simulink model "ofdm_txrx_supermimo"  (Simulink 7.1)
settings: Solver=VariableStepDiscrete  SolverMode=SingleTasking  StartTime=0.0  StopTime=simOnly_simLength  RelTol=1e-3
PostLoadFcn:
  | ofdm_rx_supermimo_init
InitFcn:
  | ofdm_rx_supermimo_init
StartFcn:
  | ofdm_rx_supermimo_init
values from neighbouring files (symbols this model uses):
  Action0_Config = 0* 31*2^18 + 0*2^24 + PHY_AUTORESPONSE_REQ_GOODHDR + PHY_AUTORESPONSE_REQ_GOODPKT + PHY_AUTORESPONSE_REQ_FLAGA    [ofdm_tx_supermimo_init.m]
  Action1_Config = 0* 62*2^18 + PHY_AUTORESPONSE_REQ_GOODHDR + PHY_AUTORESPONSE_REQ_GOODPKT    [ofdm_tx_supermimo_init.m]
  Action2_Config = 0    [ofdm_tx_supermimo_init.m]
  Action3_Config = 0    [ofdm_tx_supermimo_init.m]
  Action4_Config = 0    [ofdm_tx_supermimo_init.m]
  Action5_Config = 0    [ofdm_tx_supermimo_init.m]
  ActionID_AFTransmit = 31    [ofdm_tx_supermimo_init.m]
  ActionID_Disabled = 0    [ofdm_tx_supermimo_init.m]
  ActionID_SetFlagA = 62    [ofdm_tx_supermimo_init.m]
  ActionID_SetFlagB = 61    [ofdm_tx_supermimo_init.m]
  BER_RAM_init_size = 2048    [ofdm_tx_supermimo_init.m]
  CPLength = 16    [ofdm_tx_supermimo_init.m]
  longCorr_coef_i = [-4*real(LTS) 0]    [ofdm_rx_supermimo_init.m]
  longCorr_coef_q = [4*imag(LTS) 0]    [ofdm_rx_supermimo_init.m]
  Match0_Config = 0    [ofdm_tx_supermimo_init.m]
  Match1_Config = 0    [ofdm_tx_supermimo_init.m]
  Match2_Config = 0    [ofdm_tx_supermimo_init.m]
  Match3_Config = 0    [ofdm_tx_supermimo_init.m]
  Match4_Config = 0    [ofdm_tx_supermimo_init.m]
  Match5_Config = 0    [ofdm_tx_supermimo_init.m]
  Match6_Config = 0    [ofdm_tx_supermimo_init.m]
  Match7_Config = 0    [ofdm_tx_supermimo_init.m]
  max_CP_length = 16    [ofdm_tx_supermimo_init.m]
  max_num_baseRateSymbols = 31    [ofdm_tx_supermimo_init.m]
  max_num_subcarriers = 64    [ofdm_tx_supermimo_init.m]
  max_num_trainingSymbols = 15    [ofdm_tx_supermimo_init.m]
  max_numBytes = 16384    [ofdm_tx_supermimo_init.m]
  max_OFDM_symbols = 511    [ofdm_tx_supermimo_init.m]
  modConstellation_qam16 = .75*[1 3 -1 -3]./3    [ofdm_tx_supermimo_init.m]
  modConstellation_qam64 = 0.875*[3 1 5 7 -3 -1 -5 -7]./7    [ofdm_tx_supermimo_init.m]
  modConstellation_qpsk = [1 -1]./sqrt(2)    [ofdm_tx_supermimo_init.m]
  numBaseRateSymbols = ceil(numHeaderBytes / numBytes_BaseRateOFDMSymbol)    [ofdm_tx_supermimo_init.m]
  numSubcarriers = 64    [ofdm_tx_supermimo_init.m]
  numTrainingSymbols = 2    [ofdm_tx_supermimo_init.m]
  pilot_indicies = 7 + ( (64-21) * 2^8) + ( (64-7) * 2^16) + (21 * 2^24)    (= 356068103)    [ofdm_tx_supermimo_init.m]
  pilotValues = hex2dec('A57D') + (2^16 * hex2dec('5A82'))    [ofdm_tx_supermimo_init.m]
  pkt_crossCorr_thresh = 0.7    [ofdm_rx_supermimo_init.m]
  pkt_energy_thresh = 0    [ofdm_rx_supermimo_init.m]
  pktByteNums = numHeaderBytes +  				byteIndex_numPayloadBytes(1)*2^8 +  				byteIndex_numPayloadBytes(2)*2^16 +  				byteIndex_simpleDynModMasks * 2^24    [ofdm_rx_supermimo_init.m]
  PktDet_Delay = 58+32 +      Symbol_Timing_Offset*2^7 +      0*2^16 +      (117* 2^24)    (= 1962935642)    [ofdm_rx_supermimo_init.m]
  pktTiming_count_payloadStart = pktTiming_count_lsStart + pktTiming_ls_num*pktTiming_ls_length    [ofdm_rx_supermimo_init.m]
  preamble = preamble_scale*[shortsyms_10 longsyms_2]    [ofdm_tx_supermimo_init.m]
  preamble_ROM = preamble_I_ROM + preamble_Q_ROM*2^16    [ofdm_tx_supermimo_init.m]
  RAM_init_size = max_numBytes    (= 16384)    [ofdm_tx_supermimo_init.m]
  reg_AF_Tx_Blanking = (320+80) + ( (320+80+79)*2^16)    (= 31392144)    [ofdm_rx_supermimo_init.m]
  reg_AF_Tx_Scaling = 2944    [ofdm_rx_supermimo_init.m]
  reg_Interrupt_PktBuf_Control = reg_InterruptControl +  	1 * 2^16 +  	0 * 2^24    (= 65656)    [ofdm_rx_supermimo_init.m]
  reg_PilotCalcParams = (4 * 2^16) + (minPilotChanMag)    (= 262184.96)    [ofdm_rx_supermimo_init.m]
  regRx_chanEst_minMag = ((0.5)*2^15) + ((0.5 * 2^15)*2^16)    (= 1073758208)    [ofdm_rx_supermimo_init.m]
  regRx_coarseCalcCorrection = round((1.3e-3 - 1.2982e-3)*2^32)    [ofdm_rx_supermimo_init.m]
  regRx_pilotCalcCorrection = round(1.00115 * 2^31)    [ofdm_rx_supermimo_init.m]
  regRx_preCFOoptions = 0 * 2^0 +  	1 * 2^1 +  	0    (= 2)    [ofdm_rx_supermimo_init.m]
  rx_controlBits = 1 * 2  		 + 1 * 4  		 + 0 * 8  		 + 1 * 16 * rx_SISO_Mode  		 + 1 * 32  		 + 1 * 64  		 + 1 * 128  		 + 1 * 256  		 + 1 * 512  		 + 1 * 1024  		 + 1 * 4096  		 + 0 * 16384  		 + 0 * 32768  		 + 1 * 65536  		 + 0 * 131072  		 + 0 * 262144  		 + 1 * 524288  		 + 1 * 1048576 * tx_Alamouti_Mode  		 + 0 * 2097152           + 1 * 33554432  		 + 0 * 4194304  		 + 0 * 8388608  		 + 0 * 16777216  		 + 1 *  ...(+49 chars)    [ofdm_rx_supermimo_init.m]
  Rx_PktDet_LongCorr_Params = LongCorrCounterLoad + 0*2^8 + 0*2^16 + 255*2^24    (= 4278190325)    [ofdm_rx_supermimo_init.m]
  Rx_PktDet_LongCorrThresholds = 8000    [ofdm_rx_supermimo_init.m]
  rx_postEq_scaling = round(rxScaling*2^11) * (1 + 2^16)    [ofdm_rx_supermimo_init.m]
  RxDataScrambling_Seq = [40 198 78 63 82 173 102 245 48 111 172 115 147 230 216 93 72 65 62 2 205 242 122 90 128 83 105 97 73 10 5 252]    [ofdm_tx_supermimo_init.m]
  RxReg_FixedPktLen = 0    [ofdm_rx_supermimo_init.m]
  shortSymbol_time = shortSymbol_time(1:16).'    [ofdm_tx_supermimo_init.m]
  simOnly_numSamples = length(preamble)+( (numSubcarriers+CPLength)*(numTrainingSymbols + numBaseRateSymbols + numFullRateSymbols/2) )    [ofdm_tx_supermimo_init.m]
  train = [train train]    [ofdm_tx_supermimo_init.m]
  tx_controlBits = (antB_preambleShift * 2^4) + (2^1 * tx_Alamouti_Mode) +  	(2^0 * tx_SISO_Mode) + 4*0 + 8*1 + 0*256 + 512*0 + 1024*0 +  	0*hex2dec('1000') + 0*hex2dec('2000') + 0*hex2dec('4000') +  	1*hex2dec('8000') + 1*hex2dec('10000') + hex2dec('20000') +  	16*hex2dec('100000')    [ofdm_tx_supermimo_init.m]
  tx_delays = (50*2^0) + (0*2^8) + (30*2^12)    (= 122930)    [ofdm_tx_supermimo_init.m]
  tx_scaling = (3072) + (12288 * 2^16)    (= 805309440)    [ofdm_tx_supermimo_init.m]
  TxDataScrambling_Seq = [40 198 78 63 82 173 102 245 48 111 172 115 147 230 216 93 72 65 62 2 205 242 122 90 128 83 105 97 73 10 5 252]    [ofdm_tx_supermimo_init.m]
  txfilt_firpm = 0.95*txfilt_firpm./(max(txfilt_firpm))    [ofdm_tx_supermimo_init.m]
  txfilt_intfilt = [0 intfilt(4,4,.8)]    [ofdm_tx_supermimo_init.m]
  txReg_symbolCounts = (2^8 *numBaseRateSymbols ) + numTrainingSymbols    [ofdm_tx_supermimo_init.m]
  TxRx_FFTScaling = (3 * 2^0) +  	(2 * 2^2) +  	(1 * 2^4) +  	(1 * 2^6) +  	(1 * 2^8) +  	(0 * 2^10)    (= 347)    [ofdm_tx_supermimo_init.m]
undefined symbols (not defined in the file or in its folder): AntA, AntB, BaseRate, Down, gcbh, numBuffs, numBytes, Up, xlBlockLoadCallback, xlGetSimulinkPeriod, xlipgetpartsetting
libraries: xbsIndex_r4 x6035, simulink x4, dspsigops x4, commchan3 x2, dspsrcs4 x2, commrflib2 x2
(part 47 of 61)

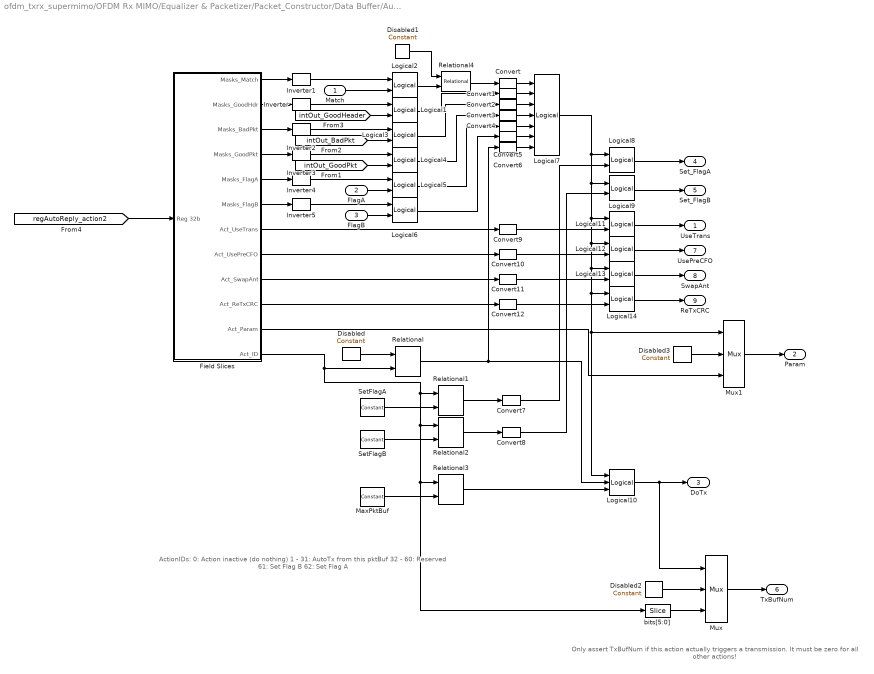
== system ofdm_txrx_supermimo/OFDM Rx MIMO/Equalizer & Packetizer/Packet_Constructor/Data Buffer/AutoResponse Processing/Action Decoding/Action2 ==
  note: ActionIDs:
      | 0: Action inactive (do nothing)
      | 1 - 31: AutoTx from this pktBuf
      | 32 - 60: Reserved
      | 61: Set Flag B
      | 62: Set Flag A
  note: Only assert TxBufNum if this action
      | actually triggers a transmission. It must
      | be zero for all other actions!
  Match : Inport
  FlagA : Inport  Port=2  OutDataType=sfix(16)  OutScaling=2^0
  FlagB : Inport  Port=3  OutDataType=sfix(16)  OutScaling=2^0
  Convert{,1..12} (13 alike) : xbsIndex_r4/Convert  arith_type=Boolean  n_bits=16  bin_pt=14  quantization=Truncate  overflow=Wrap  latency=0  dbl_ovrd=off  pipeline=off  block_type=convert
      settings: block_version=10.1.3
  Disabled : xbsIndex_r4/Constant  arith_type=Unsigned  const=ActionID_Disabled  n_bits=6  bin_pt=0  explicit_period=off  period=1  equ=P=C  opselect=C  inp2=PCIN>>17  opr=+  inp1=P  carry=CIN  dbl_ovrd=off  block_type=constant
  Disabled1 : xbsIndex_r4/Constant  arith_type=Unsigned  const=2^8-1  n_bits=8  bin_pt=0  explicit_period=off  period=1  equ=P=C  opselect=C  inp2=PCIN>>17  opr=+  inp1=P  carry=CIN  dbl_ovrd=off  block_type=constant
  Disabled2 : xbsIndex_r4/Constant  arith_type=Unsigned  const=0  n_bits=5  bin_pt=0  explicit_period=off  period=1  equ=P=C  opselect=C  inp2=PCIN>>17  opr=+  inp1=P  carry=CIN  dbl_ovrd=off  block_type=constant
  Disabled3 : xbsIndex_r4/Constant  arith_type=Unsigned  const=0  n_bits=8  bin_pt=0  explicit_period=off  period=1  equ=P=C  opselect=C  inp2=PCIN>>17  opr=+  inp1=P  carry=CIN  dbl_ovrd=off  block_type=constant
  Field Slices : Subsystem
  Inverter{,1..5} (6 alike) : xbsIndex_r4/Inverter  en=off  latency=0  dbl_ovrd=off  block_type=inv
  Logical{1..6} (6 alike) : xbsIndex_r4/Logical  logical_function=OR  inputs=2  en=off  latency=0  precision=Full  arith_type=Unsigned  n_bits=16  bin_pt=0  align_bp=on  dbl_ovrd=off  block_type=logical
  Logical10 : xbsIndex_r4/Logical  logical_function=AND  inputs=3  en=off  latency=0  precision=Full  arith_type=Unsigned  n_bits=16  bin_pt=0  align_bp=on  dbl_ovrd=off  block_type=logical
  Logical{8..9,11..14} (6 alike) : xbsIndex_r4/Logical  logical_function=AND  inputs=2  en=off  latency=0  precision=Full  arith_type=Unsigned  n_bits=16  bin_pt=0  align_bp=on  dbl_ovrd=off  block_type=logical
  Logical7 : xbsIndex_r4/Logical  logical_function=AND  inputs=7  en=off  latency=0  precision=Full  arith_type=Unsigned  n_bits=16  bin_pt=0  align_bp=on  dbl_ovrd=off  block_type=logical
  MaxPktBuf : xbsIndex_r4/Constant  arith_type=Unsigned  const=32  n_bits=6  bin_pt=0  explicit_period=off  period=1  equ=P=C  opselect=C  inp2=PCIN>>17  opr=+  inp1=P  carry=CIN  dbl_ovrd=off  block_type=constant
  Mux : xbsIndex_r4/Mux  inputs=2  en=off  latency=0  precision=Full  arith_type=Unsigned  n_bits=16  bin_pt=14  quantization=Truncate  overflow=Wrap  dbl_ovrd=off  block_type=mux
  Mux1 : xbsIndex_r4/Mux  inputs=2  en=off  latency=0  precision=Full  arith_type=Unsigned  n_bits=16  bin_pt=14  quantization=Truncate  overflow=Wrap  dbl_ovrd=off  block_type=mux
  Relational : xbsIndex_r4/Relational  mode=a!=b  en=off  latency=0  dbl_ovrd=off  block_type=relational
      settings: block_version=10.1.3
  Relational1 : xbsIndex_r4/Relational  mode=a=b  en=off  latency=0  dbl_ovrd=off  block_type=relational
      settings: block_version=10.1.3
  Relational2 : xbsIndex_r4/Relational  mode=a=b  en=off  latency=0  dbl_ovrd=off  block_type=relational
      settings: block_version=10.1.3
  Relational3 : xbsIndex_r4/Relational  mode=a<b  en=off  latency=0  dbl_ovrd=off  block_type=relational
      settings: block_version=10.1.3
  Relational4 : xbsIndex_r4/Relational  mode=a=b  en=off  latency=0  dbl_ovrd=off  block_type=relational
      settings: block_version=10.1.3
  SetFlagA : xbsIndex_r4/Constant  arith_type=Unsigned  const=ActionID_SetFlagA  n_bits=6  bin_pt=0  explicit_period=off  period=1  equ=P=C  opselect=C  inp2=PCIN>>17  opr=+  inp1=P  carry=CIN  dbl_ovrd=off  block_type=constant
  SetFlagB : xbsIndex_r4/Constant  arith_type=Unsigned  const=ActionID_SetFlagB  n_bits=6  bin_pt=0  explicit_period=off  period=1  equ=P=C  opselect=C  inp2=PCIN>>17  opr=+  inp1=P  carry=CIN  dbl_ovrd=off  block_type=constant
  bits[5:0] : xbsIndex_r4/Slice  nbits=5  boolean_output=off  mode=Lower Bit Location + Width  bit1=0  base1=MSB of Input  bit0=0  base0=LSB of Input  dbl_ovrd=off  block_type=slice
      settings: infoedit="Extracts a given range of bits from each input sample and presents it at the output.  The output type is ordinarily unsigned with binary point at zero, but can be Boolean when the slice is one bit wide.<P><P>Hardware notes: In hardware this block costs nothing."  block_version=VER_STRING_GOES_HERE
  UseTrans : Outport
  Param : Outport  Port=2
  DoTx : Outport  Port=3
  Set_FlagA : Outport  Port=4
  Set_FlagB : Outport  Port=5
  TxBufNum : Outport  Port=6
  UsePreCFO : Outport  Port=7
  SwapAnt : Outport  Port=8
  ReTxCRC : Outport  Port=9
  wires:
  Mux/1 -> TxBufNum/1
  Disabled2/1 -> Mux/2
  bits[5:0]/1 -> Mux/3
  FlagB/1 -> Logical6/2
  FlagA/1 -> Logical5/2
  Field Slices/11(Act_Param) -> Mux1/3
  Field Slices/7(Act_UseTrans) -> Convert9/1
  Match/1 -> Logical2/2
  Logical10/1 -> Mux/1, DoTx/1
  Relational3/1 -> Logical10/3
  Relational2/1 -> Convert8/1
  Relational1/1 -> Convert7/1
  Logical9/1 -> Set_FlagB/1
  Logical7/1 -> Logical9/1, Logical11/1, Logical12/1, Logical13/1, Logical10/1, Mux1/1, Logical14/1, Logical8/1
  Logical8/1 -> Set_FlagA/1
  Relational/1 -> Convert6/1, Logical10/2
  Logical6/1 -> Convert5/1
  Logical5/1 -> Convert4/1
  Logical4/1 -> Convert3/1
  Logical3/1 -> Convert2/1
  Logical1/1 -> Convert1/1
  Logical2/1 -> Relational4/2
  MaxPktBuf/1 -> Relational3/2
  SetFlagB/1 -> Relational2/2
  SetFlagA/1 -> Relational1/2
  Field Slices/12(Act_ID) -> Relational2/1, Relational3/1, bits[5:0]/1, Relational1/1, Relational/2
  Inverter5/1 -> Logical6/1
  Field Slices/6(Masks_FlagB) -> Inverter5/1
  Inverter4/1 -> Logical5/1
  Field Slices/5(Masks_FlagA) -> Inverter4/1
  Field Slices/4(Masks_GoodPkt) -> Inverter3/1
  [intOut_GoodPkt] -> Logical4/2
  Inverter3/1 -> Logical4/1
  [intOut_BadPkt] -> Logical3/2
  Inverter2/1 -> Logical3/1
  Field Slices/3(Masks_BadPkt) -> Inverter2/1
  Inverter1/1 -> Logical2/1
  [intOut_GoodHeader] -> Logical1/2
  Inverter/1 -> Logical1/1
  Field Slices/2(Masks_GoodHdr) -> Inverter/1
  Field Slices/1(Masks_Match) -> Inverter1/1
  [regAutoReply_action2] -> Field Slices/1(Reg 32b)
  Convert/1 -> Logical7/1
  Convert1/1 -> Logical7/2
  Convert2/1 -> Logical7/3
  Convert3/1 -> Logical7/4
  Convert4/1 -> Logical7/5
  Convert5/1 -> Logical7/6
  Convert6/1 -> Logical7/7
  Convert7/1 -> Logical8/2
  Convert8/1 -> Logical9/2
  Relational4/1 -> Convert/1
  Disabled1/1 -> Relational4/1
  Logical11/1 -> UseTrans/1
  Mux1/1 -> Param/1
  Disabled3/1 -> Mux1/2
  Convert9/1 -> Logical11/2
  Field Slices/8(Act_UsePreCFO) -> Convert10/1
  Convert10/1 -> Logical12/2
  Logical12/1 -> UsePreCFO/1
  Logical13/1 -> SwapAnt/1
  Field Slices/9(Act_SwapAnt) -> Convert11/1
  Convert11/1 -> Logical13/2
  Disabled/1 -> Relational/1
  Field Slices/10(Act_ReTxCRC) -> Convert12/1
  Convert12/1 -> Logical14/2
  Logical14/1 -> ReTxCRC/1

== system ofdm_txrx_supermimo/OFDM Rx MIMO/Equalizer & Packetizer/Packet_Constructor/Data Buffer/AutoResponse Processing/Action Decoding/Action3 ==
  note: Only assert TxBufNum if this action
      | actually triggers a transmission. It must
      | be zero for all other actions!
  note: ActionIDs:
      | 0: Action inactive (do nothing)
      | 1 - 31: AutoTx from this pktBuf
      | 32 - 60: Reserved
      | 61: Set Flag B
      | 62: Set Flag A
  Match : Inport
  FlagA : Inport  Port=2  OutDataType=sfix(16)  OutScaling=2^0
  FlagB : Inport  Port=3  OutDataType=sfix(16)  OutScaling=2^0
  Convert{,1..12} (13 alike) : xbsIndex_r4/Convert  arith_type=Boolean  n_bits=16  bin_pt=14  quantization=Truncate  overflow=Wrap  latency=0  dbl_ovrd=off  pipeline=off  block_type=convert
      settings: block_version=10.1.3
  Disabled : xbsIndex_r4/Constant  arith_type=Unsigned  const=ActionID_Disabled  n_bits=6  bin_pt=0  explicit_period=off  period=1  equ=P=C  opselect=C  inp2=PCIN>>17  opr=+  inp1=P  carry=CIN  dbl_ovrd=off  block_type=constant
  Disabled1 : xbsIndex_r4/Constant  arith_type=Unsigned  const=2^8-1  n_bits=8  bin_pt=0  explicit_period=off  period=1  equ=P=C  opselect=C  inp2=PCIN>>17  opr=+  inp1=P  carry=CIN  dbl_ovrd=off  block_type=constant
  Disabled2 : xbsIndex_r4/Constant  arith_type=Unsigned  const=0  n_bits=5  bin_pt=0  explicit_period=off  period=1  equ=P=C  opselect=C  inp2=PCIN>>17  opr=+  inp1=P  carry=CIN  dbl_ovrd=off  block_type=constant
  Disabled3 : xbsIndex_r4/Constant  arith_type=Unsigned  const=0  n_bits=8  bin_pt=0  explicit_period=off  period=1  equ=P=C  opselect=C  inp2=PCIN>>17  opr=+  inp1=P  carry=CIN  dbl_ovrd=off  block_type=constant
  Field Slices : Subsystem
  Inverter{,1..5} (6 alike) : xbsIndex_r4/Inverter  en=off  latency=0  dbl_ovrd=off  block_type=inv
  Logical{1..6} (6 alike) : xbsIndex_r4/Logical  logical_function=OR  inputs=2  en=off  latency=0  precision=Full  arith_type=Unsigned  n_bits=16  bin_pt=0  align_bp=on  dbl_ovrd=off  block_type=logical
  Logical10 : xbsIndex_r4/Logical  logical_function=AND  inputs=3  en=off  latency=0  precision=Full  arith_type=Unsigned  n_bits=16  bin_pt=0  align_bp=on  dbl_ovrd=off  block_type=logical
  Logical{8..9,11..14} (6 alike) : xbsIndex_r4/Logical  logical_function=AND  inputs=2  en=off  latency=0  precision=Full  arith_type=Unsigned  n_bits=16  bin_pt=0  align_bp=on  dbl_ovrd=off  block_type=logical
  Logical7 : xbsIndex_r4/Logical  logical_function=AND  inputs=7  en=off  latency=0  precision=Full  arith_type=Unsigned  n_bits=16  bin_pt=0  align_bp=on  dbl_ovrd=off  block_type=logical
  MaxPktBuf : xbsIndex_r4/Constant  arith_type=Unsigned  const=32  n_bits=6  bin_pt=0  explicit_period=off  period=1  equ=P=C  opselect=C  inp2=PCIN>>17  opr=+  inp1=P  carry=CIN  dbl_ovrd=off  block_type=constant
  Mux : xbsIndex_r4/Mux  inputs=2  en=off  latency=0  precision=Full  arith_type=Unsigned  n_bits=16  bin_pt=14  quantization=Truncate  overflow=Wrap  dbl_ovrd=off  block_type=mux
  Mux1 : xbsIndex_r4/Mux  inputs=2  en=off  latency=0  precision=Full  arith_type=Unsigned  n_bits=16  bin_pt=14  quantization=Truncate  overflow=Wrap  dbl_ovrd=off  block_type=mux
  Relational : xbsIndex_r4/Relational  mode=a!=b  en=off  latency=0  dbl_ovrd=off  block_type=relational
      settings: block_version=10.1.3
  Relational1 : xbsIndex_r4/Relational  mode=a=b  en=off  latency=0  dbl_ovrd=off  block_type=relational
      settings: block_version=10.1.3
  Relational2 : xbsIndex_r4/Relational  mode=a=b  en=off  latency=0  dbl_ovrd=off  block_type=relational
      settings: block_version=10.1.3
  Relational3 : xbsIndex_r4/Relational  mode=a<b  en=off  latency=0  dbl_ovrd=off  block_type=relational
      settings: block_version=10.1.3
  Relational4 : xbsIndex_r4/Relational  mode=a=b  en=off  latency=0  dbl_ovrd=off  block_type=relational
      settings: block_version=10.1.3
  SetFlagA : xbsIndex_r4/Constant  arith_type=Unsigned  const=ActionID_SetFlagA  n_bits=6  bin_pt=0  explicit_period=off  period=1  equ=P=C  opselect=C  inp2=PCIN>>17  opr=+  inp1=P  carry=CIN  dbl_ovrd=off  block_type=constant
  SetFlagB : xbsIndex_r4/Constant  arith_type=Unsigned  const=ActionID_SetFlagB  n_bits=6  bin_pt=0  explicit_period=off  period=1  equ=P=C  opselect=C  inp2=PCIN>>17  opr=+  inp1=P  carry=CIN  dbl_ovrd=off  block_type=constant
  bits[5:0] : xbsIndex_r4/Slice  nbits=5  boolean_output=off  mode=Lower Bit Location + Width  bit1=0  base1=MSB of Input  bit0=0  base0=LSB of Input  dbl_ovrd=off  block_type=slice
      settings: infoedit="Extracts a given range of bits from each input sample and presents it at the output.  The output type is ordinarily unsigned with binary point at zero, but can be Boolean when the slice is one bit wide.<P><P>Hardware notes: In hardware this block costs nothing."  block_version=VER_STRING_GOES_HERE
  UseTrans : Outport
  Param : Outport  Port=2
  DoTx : Outport  Port=3
  Set_FlagA : Outport  Port=4
  Set_FlagB : Outport  Port=5
  TxBufNum : Outport  Port=6
  UsePreCFO : Outport  Port=7
  SwapAnt : Outport  Port=8
  ReTxCRC : Outport  Port=9
  wires:
  Logical14/1 -> ReTxCRC/1
  Convert12/1 -> Logical14/2
  Field Slices/10(Act_ReTxCRC) -> Convert12/1
  Disabled/1 -> Relational/1
  Convert11/1 -> Logical13/2
  Field Slices/9(Act_SwapAnt) -> Convert11/1
  Logical13/1 -> SwapAnt/1
  Logical12/1 -> UsePreCFO/1
  Convert10/1 -> Logical12/2
  Field Slices/8(Act_UsePreCFO) -> Convert10/1
  Convert9/1 -> Logical11/2
  Disabled3/1 -> Mux1/2
  Mux1/1 -> Param/1
  Logical11/1 -> UseTrans/1
  Disabled1/1 -> Relational4/1
  Relational4/1 -> Convert/1
  Convert8/1 -> Logical9/2
  Convert7/1 -> Logical8/2
  Convert6/1 -> Logical7/7
  Convert5/1 -> Logical7/6
  Convert4/1 -> Logical7/5
  Convert3/1 -> Logical7/4
  Convert2/1 -> Logical7/3
  Convert1/1 -> Logical7/2
  Convert/1 -> Logical7/1
  [regAutoReply_action3] -> Field Slices/1(Reg 32b)
  Field Slices/1(Masks_Match) -> Inverter1/1
  Field Slices/2(Masks_GoodHdr) -> Inverter/1
  Inverter/1 -> Logical1/1
  [intOut_GoodHeader] -> Logical1/2
  Inverter1/1 -> Logical2/1
  Field Slices/3(Masks_BadPkt) -> Inverter2/1
  Inverter2/1 -> Logical3/1
  [intOut_BadPkt] -> Logical3/2
  Inverter3/1 -> Logical4/1
  [intOut_GoodPkt] -> Logical4/2
  Field Slices/4(Masks_GoodPkt) -> Inverter3/1
  Field Slices/5(Masks_FlagA) -> Inverter4/1
  Inverter4/1 -> Logical5/1
  Field Slices/6(Masks_FlagB) -> Inverter5/1
  Inverter5/1 -> Logical6/1
  Field Slices/12(Act_ID) -> Relational/2, Relational1/1, bits[5:0]/1, Relational3/1, Relational2/1
  SetFlagA/1 -> Relational1/2
  SetFlagB/1 -> Relational2/2
  MaxPktBuf/1 -> Relational3/2
  Logical2/1 -> Relational4/2
  Logical1/1 -> Convert1/1
  Logical3/1 -> Convert2/1
  Logical4/1 -> Convert3/1
  Logical5/1 -> Convert4/1
  Logical6/1 -> Convert5/1
  Relational/1 -> Logical10/2, Convert6/1
  Logical8/1 -> Set_FlagA/1
  Logical7/1 -> Logical8/1, Logical14/1, Mux1/1, Logical10/1, Logical13/1, Logical12/1, Logical11/1, Logical9/1
  Logical9/1 -> Set_FlagB/1
  Relational1/1 -> Convert7/1
  Relational2/1 -> Convert8/1
  Relational3/1 -> Logical10/3
  Logical10/1 -> DoTx/1, Mux/1
  Match/1 -> Logical2/2
  Field Slices/7(Act_UseTrans) -> Convert9/1
  Field Slices/11(Act_Param) -> Mux1/3
  FlagA/1 -> Logical5/2
  FlagB/1 -> Logical6/2
  bits[5:0]/1 -> Mux/3
  Disabled2/1 -> Mux/2
  Mux/1 -> TxBufNum/1

== system ofdm_txrx_supermimo/OFDM Rx MIMO/Equalizer & Packetizer/Packet_Constructor/Data Buffer/AutoResponse Processing/Action Decoding/Action4 ==
  note: ActionIDs:
      | 0: Action inactive (do nothing)
      | 1 - 31: AutoTx from this pktBuf
      | 32 - 60: Reserved
      | 61: Set Flag B
      | 62: Set Flag A
  note: Only assert TxBufNum if this action
      | actually triggers a transmission. It must
      | be zero for all other actions!
  Match : Inport
  FlagA : Inport  Port=2  OutDataType=sfix(16)  OutScaling=2^0
  FlagB : Inport  Port=3  OutDataType=sfix(16)  OutScaling=2^0
  Convert{,1..12} (13 alike) : xbsIndex_r4/Convert  arith_type=Boolean  n_bits=16  bin_pt=14  quantization=Truncate  overflow=Wrap  latency=0  dbl_ovrd=off  pipeline=off  block_type=convert
      settings: block_version=10.1.3
  Disabled : xbsIndex_r4/Constant  arith_type=Unsigned  const=ActionID_Disabled  n_bits=6  bin_pt=0  explicit_period=off  period=1  equ=P=C  opselect=C  inp2=PCIN>>17  opr=+  inp1=P  carry=CIN  dbl_ovrd=off  block_type=constant
  Disabled1 : xbsIndex_r4/Constant  arith_type=Unsigned  const=2^8-1  n_bits=8  bin_pt=0  explicit_period=off  period=1  equ=P=C  opselect=C  inp2=PCIN>>17  opr=+  inp1=P  carry=CIN  dbl_ovrd=off  block_type=constant
  Disabled2 : xbsIndex_r4/Constant  arith_type=Unsigned  const=0  n_bits=5  bin_pt=0  explicit_period=off  period=1  equ=P=C  opselect=C  inp2=PCIN>>17  opr=+  inp1=P  carry=CIN  dbl_ovrd=off  block_type=constant
  Disabled3 : xbsIndex_r4/Constant  arith_type=Unsigned  const=0  n_bits=8  bin_pt=0  explicit_period=off  period=1  equ=P=C  opselect=C  inp2=PCIN>>17  opr=+  inp1=P  carry=CIN  dbl_ovrd=off  block_type=constant
  Field Slices : Subsystem
  Inverter{,1..5} (6 alike) : xbsIndex_r4/Inverter  en=off  latency=0  dbl_ovrd=off  block_type=inv
  Logical{1..6} (6 alike) : xbsIndex_r4/Logical  logical_function=OR  inputs=2  en=off  latency=0  precision=Full  arith_type=Unsigned  n_bits=16  bin_pt=0  align_bp=on  dbl_ovrd=off  block_type=logical
  Logical10 : xbsIndex_r4/Logical  logical_function=AND  inputs=3  en=off  latency=0  precision=Full  arith_type=Unsigned  n_bits=16  bin_pt=0  align_bp=on  dbl_ovrd=off  block_type=logical
  Logical{8..9,11..14} (6 alike) : xbsIndex_r4/Logical  logical_function=AND  inputs=2  en=off  latency=0  precision=Full  arith_type=Unsigned  n_bits=16  bin_pt=0  align_bp=on  dbl_ovrd=off  block_type=logical
  Logical7 : xbsIndex_r4/Logical  logical_function=AND  inputs=7  en=off  latency=0  precision=Full  arith_type=Unsigned  n_bits=16  bin_pt=0  align_bp=on  dbl_ovrd=off  block_type=logical
  MaxPktBuf : xbsIndex_r4/Constant  arith_type=Unsigned  const=32  n_bits=6  bin_pt=0  explicit_period=off  period=1  equ=P=C  opselect=C  inp2=PCIN>>17  opr=+  inp1=P  carry=CIN  dbl_ovrd=off  block_type=constant
  Mux : xbsIndex_r4/Mux  inputs=2  en=off  latency=0  precision=Full  arith_type=Unsigned  n_bits=16  bin_pt=14  quantization=Truncate  overflow=Wrap  dbl_ovrd=off  block_type=mux
  Mux1 : xbsIndex_r4/Mux  inputs=2  en=off  latency=0  precision=Full  arith_type=Unsigned  n_bits=16  bin_pt=14  quantization=Truncate  overflow=Wrap  dbl_ovrd=off  block_type=mux
  Relational : xbsIndex_r4/Relational  mode=a!=b  en=off  latency=0  dbl_ovrd=off  block_type=relational
      settings: block_version=10.1.3
  Relational1 : xbsIndex_r4/Relational  mode=a=b  en=off  latency=0  dbl_ovrd=off  block_type=relational
      settings: block_version=10.1.3
  Relational2 : xbsIndex_r4/Relational  mode=a=b  en=off  latency=0  dbl_ovrd=off  block_type=relational
      settings: block_version=10.1.3
  Relational3 : xbsIndex_r4/Relational  mode=a<b  en=off  latency=0  dbl_ovrd=off  block_type=relational
      settings: block_version=10.1.3
  Relational4 : xbsIndex_r4/Relational  mode=a=b  en=off  latency=0  dbl_ovrd=off  block_type=relational
      settings: block_version=10.1.3
  SetFlagA : xbsIndex_r4/Constant  arith_type=Unsigned  const=ActionID_SetFlagA  n_bits=6  bin_pt=0  explicit_period=off  period=1  equ=P=C  opselect=C  inp2=PCIN>>17  opr=+  inp1=P  carry=CIN  dbl_ovrd=off  block_type=constant
  SetFlagB : xbsIndex_r4/Constant  arith_type=Unsigned  const=ActionID_SetFlagB  n_bits=6  bin_pt=0  explicit_period=off  period=1  equ=P=C  opselect=C  inp2=PCIN>>17  opr=+  inp1=P  carry=CIN  dbl_ovrd=off  block_type=constant
  bits[5:0] : xbsIndex_r4/Slice  nbits=5  boolean_output=off  mode=Lower Bit Location + Width  bit1=0  base1=MSB of Input  bit0=0  base0=LSB of Input  dbl_ovrd=off  block_type=slice
      settings: infoedit="Extracts a given range of bits from each input sample and presents it at the output.  The output type is ordinarily unsigned with binary point at zero, but can be Boolean when the slice is one bit wide.<P><P>Hardware notes: In hardware this block costs nothing."  block_version=VER_STRING_GOES_HERE
  UseTrans : Outport
  Param : Outport  Port=2
  DoTx : Outport  Port=3
  Set_FlagA : Outport  Port=4
  Set_FlagB : Outport  Port=5
  TxBufNum : Outport  Port=6
  UsePreCFO : Outport  Port=7
  SwapAnt : Outport  Port=8
  ReTxCRC : Outport  Port=9
  wires:
  Mux/1 -> TxBufNum/1
  Disabled2/1 -> Mux/2
  bits[5:0]/1 -> Mux/3
  FlagB/1 -> Logical6/2
  FlagA/1 -> Logical5/2
  Field Slices/11(Act_Param) -> Mux1/3
  Field Slices/7(Act_UseTrans) -> Convert9/1
  Match/1 -> Logical2/2
  Logical10/1 -> Mux/1, DoTx/1
  Relational3/1 -> Logical10/3
  Relational2/1 -> Convert8/1
  Relational1/1 -> Convert7/1
  Logical9/1 -> Set_FlagB/1
  Logical7/1 -> Logical9/1, Logical11/1, Logical12/1, Logical13/1, Logical10/1, Mux1/1, Logical14/1, Logical8/1
  Logical8/1 -> Set_FlagA/1
  Relational/1 -> Convert6/1, Logical10/2
  Logical6/1 -> Convert5/1
  Logical5/1 -> Convert4/1
  Logical4/1 -> Convert3/1
  Logical3/1 -> Convert2/1
  Logical1/1 -> Convert1/1
  Logical2/1 -> Relational4/2
  MaxPktBuf/1 -> Relational3/2
  SetFlagB/1 -> Relational2/2
  SetFlagA/1 -> Relational1/2
  Field Slices/12(Act_ID) -> Relational2/1, Relational3/1, bits[5:0]/1, Relational1/1, Relational/2
  Inverter5/1 -> Logical6/1
  Field Slices/6(Masks_FlagB) -> Inverter5/1
  Inverter4/1 -> Logical5/1
  Field Slices/5(Masks_FlagA) -> Inverter4/1
  Field Slices/4(Masks_GoodPkt) -> Inverter3/1
  [intOut_GoodPkt] -> Logical4/2
  Inverter3/1 -> Logical4/1
  [intOut_BadPkt] -> Logical3/2
  Inverter2/1 -> Logical3/1
  Field Slices/3(Masks_BadPkt) -> Inverter2/1
  Inverter1/1 -> Logical2/1
  [intOut_GoodHeader] -> Logical1/2
  Inverter/1 -> Logical1/1
  Field Slices/2(Masks_GoodHdr) -> Inverter/1
  Field Slices/1(Masks_Match) -> Inverter1/1
  [regAutoReply_action4] -> Field Slices/1(Reg 32b)
  Convert/1 -> Logical7/1
  Convert1/1 -> Logical7/2
  Convert2/1 -> Logical7/3
  Convert3/1 -> Logical7/4
  Convert4/1 -> Logical7/5
  Convert5/1 -> Logical7/6
  Convert6/1 -> Logical7/7
  Convert7/1 -> Logical8/2
  Convert8/1 -> Logical9/2
  Relational4/1 -> Convert/1
  Disabled1/1 -> Relational4/1
  Logical11/1 -> UseTrans/1
  Mux1/1 -> Param/1
  Disabled3/1 -> Mux1/2
  Convert9/1 -> Logical11/2
  Field Slices/8(Act_UsePreCFO) -> Convert10/1
  Convert10/1 -> Logical12/2
  Logical12/1 -> UsePreCFO/1
  Logical13/1 -> SwapAnt/1
  Field Slices/9(Act_SwapAnt) -> Convert11/1
  Convert11/1 -> Logical13/2
  Disabled/1 -> Relational/1
  Field Slices/10(Act_ReTxCRC) -> Convert12/1
  Convert12/1 -> Logical14/2
  Logical14/1 -> ReTxCRC/1

== system ofdm_txrx_supermimo/OFDM Rx MIMO/Equalizer & Packetizer/Packet_Constructor/Data Buffer/AutoResponse Processing/Action Decoding/Action5 ==
  note: Only assert TxBufNum if this action
      | actually triggers a transmission. It must
      | be zero for all other actions!
  note: ActionIDs:
      | 0: Action inactive (do nothing)
      | 1 - 31: AutoTx from this pktBuf
      | 32 - 60: Reserved
      | 61: Set Flag B
      | 62: Set Flag A
  Match : Inport
  FlagA : Inport  Port=2  OutDataType=sfix(16)  OutScaling=2^0
  FlagB : Inport  Port=3  OutDataType=sfix(16)  OutScaling=2^0
  Convert{,1..12} (13 alike) : xbsIndex_r4/Convert  arith_type=Boolean  n_bits=16  bin_pt=14  quantization=Truncate  overflow=Wrap  latency=0  dbl_ovrd=off  pipeline=off  block_type=convert
      settings: block_version=10.1.3
  Disabled : xbsIndex_r4/Constant  arith_type=Unsigned  const=ActionID_Disabled  n_bits=6  bin_pt=0  explicit_period=off  period=1  equ=P=C  opselect=C  inp2=PCIN>>17  opr=+  inp1=P  carry=CIN  dbl_ovrd=off  block_type=constant
  Disabled1 : xbsIndex_r4/Constant  arith_type=Unsigned  const=2^8-1  n_bits=8  bin_pt=0  explicit_period=off  period=1  equ=P=C  opselect=C  inp2=PCIN>>17  opr=+  inp1=P  carry=CIN  dbl_ovrd=off  block_type=constant
  Disabled2 : xbsIndex_r4/Constant  arith_type=Unsigned  const=0  n_bits=5  bin_pt=0  explicit_period=off  period=1  equ=P=C  opselect=C  inp2=PCIN>>17  opr=+  inp1=P  carry=CIN  dbl_ovrd=off  block_type=constant
  Disabled3 : xbsIndex_r4/Constant  arith_type=Unsigned  const=0  n_bits=8  bin_pt=0  explicit_period=off  period=1  equ=P=C  opselect=C  inp2=PCIN>>17  opr=+  inp1=P  carry=CIN  dbl_ovrd=off  block_type=constant
  Field Slices : Subsystem
  Inverter{,1..5} (6 alike) : xbsIndex_r4/Inverter  en=off  latency=0  dbl_ovrd=off  block_type=inv
  Logical{1..6} (6 alike) : xbsIndex_r4/Logical  logical_function=OR  inputs=2  en=off  latency=0  precision=Full  arith_type=Unsigned  n_bits=16  bin_pt=0  align_bp=on  dbl_ovrd=off  block_type=logical
  Logical10 : xbsIndex_r4/Logical  logical_function=AND  inputs=3  en=off  latency=0  precision=Full  arith_type=Unsigned  n_bits=16  bin_pt=0  align_bp=on  dbl_ovrd=off  block_type=logical
  Logical{8..9,11..14} (6 alike) : xbsIndex_r4/Logical  logical_function=AND  inputs=2  en=off  latency=0  precision=Full  arith_type=Unsigned  n_bits=16  bin_pt=0  align_bp=on  dbl_ovrd=off  block_type=logical
  Logical7 : xbsIndex_r4/Logical  logical_function=AND  inputs=7  en=off  latency=0  precision=Full  arith_type=Unsigned  n_bits=16  bin_pt=0  align_bp=on  dbl_ovrd=off  block_type=logical
  MaxPktBuf : xbsIndex_r4/Constant  arith_type=Unsigned  const=32  n_bits=6  bin_pt=0  explicit_period=off  period=1  equ=P=C  opselect=C  inp2=PCIN>>17  opr=+  inp1=P  carry=CIN  dbl_ovrd=off  block_type=constant
  Mux : xbsIndex_r4/Mux  inputs=2  en=off  latency=0  precision=Full  arith_type=Unsigned  n_bits=16  bin_pt=14  quantization=Truncate  overflow=Wrap  dbl_ovrd=off  block_type=mux
  Mux1 : xbsIndex_r4/Mux  inputs=2  en=off  latency=0  precision=Full  arith_type=Unsigned  n_bits=16  bin_pt=14  quantization=Truncate  overflow=Wrap  dbl_ovrd=off  block_type=mux
  Relational : xbsIndex_r4/Relational  mode=a!=b  en=off  latency=0  dbl_ovrd=off  block_type=relational
      settings: block_version=10.1.3
  Relational1 : xbsIndex_r4/Relational  mode=a=b  en=off  latency=0  dbl_ovrd=off  block_type=relational
      settings: block_version=10.1.3
  Relational2 : xbsIndex_r4/Relational  mode=a=b  en=off  latency=0  dbl_ovrd=off  block_type=relational
      settings: block_version=10.1.3
  Relational3 : xbsIndex_r4/Relational  mode=a<b  en=off  latency=0  dbl_ovrd=off  block_type=relational
      settings: block_version=10.1.3
  Relational4 : xbsIndex_r4/Relational  mode=a=b  en=off  latency=0  dbl_ovrd=off  block_type=relational
      settings: block_version=10.1.3
  SetFlagA : xbsIndex_r4/Constant  arith_type=Unsigned  const=ActionID_SetFlagA  n_bits=6  bin_pt=0  explicit_period=off  period=1  equ=P=C  opselect=C  inp2=PCIN>>17  opr=+  inp1=P  carry=CIN  dbl_ovrd=off  block_type=constant
  SetFlagB : xbsIndex_r4/Constant  arith_type=Unsigned  const=ActionID_SetFlagB  n_bits=6  bin_pt=0  explicit_period=off  period=1  equ=P=C  opselect=C  inp2=PCIN>>17  opr=+  inp1=P  carry=CIN  dbl_ovrd=off  block_type=constant
  bits[5:0] : xbsIndex_r4/Slice  nbits=5  boolean_output=off  mode=Lower Bit Location + Width  bit1=0  base1=MSB of Input  bit0=0  base0=LSB of Input  dbl_ovrd=off  block_type=slice
      settings: infoedit="Extracts a given range of bits from each input sample and presents it at the output.  The output type is ordinarily unsigned with binary point at zero, but can be Boolean when the slice is one bit wide.<P><P>Hardware notes: In hardware this block costs nothing."  block_version=VER_STRING_GOES_HERE
  UseTrans : Outport
  Param : Outport  Port=2
  DoTx : Outport  Port=3
  Set_FlagA : Outport  Port=4
  Set_FlagB : Outport  Port=5
  TxBufNum : Outport  Port=6
  UsePreCFO : Outport  Port=7
  SwapAnt : Outport  Port=8
  ReTxCRC : Outport  Port=9
  wires:
  Logical14/1 -> ReTxCRC/1
  Convert12/1 -> Logical14/2
  Field Slices/10(Act_ReTxCRC) -> Convert12/1
  Disabled/1 -> Relational/1
  Convert11/1 -> Logical13/2
  Field Slices/9(Act_SwapAnt) -> Convert11/1
  Logical13/1 -> SwapAnt/1
  Logical12/1 -> UsePreCFO/1
  Convert10/1 -> Logical12/2
  Field Slices/8(Act_UsePreCFO) -> Convert10/1
  Convert9/1 -> Logical11/2
  Disabled3/1 -> Mux1/2
  Mux1/1 -> Param/1
  Logical11/1 -> UseTrans/1
  Disabled1/1 -> Relational4/1
  Relational4/1 -> Convert/1
  Convert8/1 -> Logical9/2
  Convert7/1 -> Logical8/2
  Convert6/1 -> Logical7/7
  Convert5/1 -> Logical7/6
  Convert4/1 -> Logical7/5
  Convert3/1 -> Logical7/4
  Convert2/1 -> Logical7/3
  Convert1/1 -> Logical7/2
  Convert/1 -> Logical7/1
  [regAutoReply_action5] -> Field Slices/1(Reg 32b)
  Field Slices/1(Masks_Match) -> Inverter1/1
  Field Slices/2(Masks_GoodHdr) -> Inverter/1
  Inverter/1 -> Logical1/1
  [intOut_GoodHeader] -> Logical1/2
  Inverter1/1 -> Logical2/1
  Field Slices/3(Masks_BadPkt) -> Inverter2/1
  Inverter2/1 -> Logical3/1
  [intOut_BadPkt] -> Logical3/2
  Inverter3/1 -> Logical4/1
  [intOut_GoodPkt] -> Logical4/2
  Field Slices/4(Masks_GoodPkt) -> Inverter3/1
  Field Slices/5(Masks_FlagA) -> Inverter4/1
  Inverter4/1 -> Logical5/1
  Field Slices/6(Masks_FlagB) -> Inverter5/1
  Inverter5/1 -> Logical6/1
  Field Slices/12(Act_ID) -> Relational/2, Relational1/1, bits[5:0]/1, Relational3/1, Relational2/1
  SetFlagA/1 -> Relational1/2
  SetFlagB/1 -> Relational2/2
  MaxPktBuf/1 -> Relational3/2
  Logical2/1 -> Relational4/2
  Logical1/1 -> Convert1/1
  Logical3/1 -> Convert2/1
  Logical4/1 -> Convert3/1
  Logical5/1 -> Convert4/1
  Logical6/1 -> Convert5/1
  Relational/1 -> Logical10/2, Convert6/1
  Logical8/1 -> Set_FlagA/1
  Logical7/1 -> Logical8/1, Logical14/1, Mux1/1, Logical10/1, Logical13/1, Logical12/1, Logical11/1, Logical9/1
  Logical9/1 -> Set_FlagB/1
  Relational1/1 -> Convert7/1
  Relational2/1 -> Convert8/1
  Relational3/1 -> Logical10/3
  Logical10/1 -> DoTx/1, Mux/1
  Match/1 -> Logical2/2
  Field Slices/7(Act_UseTrans) -> Convert9/1
  Field Slices/11(Act_Param) -> Mux1/3
  FlagA/1 -> Logical5/2
  FlagB/1 -> Logical6/2
  bits[5:0]/1 -> Mux/3
  Disabled2/1 -> Mux/2
  Mux/1 -> TxBufNum/1

== system ofdm_txrx_supermimo/OFDM Rx MIMO/Equalizer & Packetizer/Packet_Constructor/Data Buffer/AutoResponse Processing/AutoResponse I/O/S-R Latch ==
  S : Inport  OutDataType=sfix(16)  OutScaling=2^0
  R : Inport  Port=2  OutDataType=sfix(16)  OutScaling=2^0
  Constant1 : xbsIndex_r4/Constant  arith_type=Boolean  const=1  n_bits=1  bin_pt=0  explicit_period=off  period=1  equ=P=C  opselect=C  inp2=PCIN>>17  opr=+  inp1=P  carry=CIN  dbl_ovrd=off  block_type=constant
      settings: block_version=VER_STRING_GOES_HERE
  Convert : xbsIndex_r4/Convert  arith_type=Boolean  n_bits=16  bin_pt=14  quantization=Truncate  overflow=Wrap  latency=0  dbl_ovrd=off  pipeline=off  block_type=convert
      settings: block_version=10.1.3
  Convert1 : xbsIndex_r4/Convert  arith_type=Boolean  n_bits=16  bin_pt=14  quantization=Truncate  overflow=Wrap  latency=0  dbl_ovrd=off  pipeline=off  block_type=convert
      settings: block_version=10.1.3
  Register2 : xbsIndex_r4/Register  init=0  rst=on  en=on  dbl_ovrd=off  block_type=register
      settings: xl_area=[1 1 0 0 0 0 0]  block_version=VER_STRING_GOES_HERE
  Q : Outport  OutDataType=sfix(16)  OutScaling=2^0
  wires:
  S/1 -> Convert1/1
  Register2/1 -> Q/1
  R/1 -> Convert/1
  Constant1/1 -> Register2/1
  Convert/1 -> Register2/2
  Convert1/1 -> Register2/3

== system ofdm_txrx_supermimo/OFDM Rx MIMO/Equalizer & Packetizer/Packet_Constructor/Data Buffer/AutoResponse Processing/AutoResponse I/O/S-R Latch1 ==
  S : Inport  OutDataType=sfix(16)  OutScaling=2^0
  R : Inport  Port=2  OutDataType=sfix(16)  OutScaling=2^0
  Constant1 : xbsIndex_r4/Constant  arith_type=Boolean  const=1  n_bits=1  bin_pt=0  explicit_period=off  period=1  equ=P=C  opselect=C  inp2=PCIN>>17  opr=+  inp1=P  carry=CIN  dbl_ovrd=off  block_type=constant
      settings: block_version=VER_STRING_GOES_HERE
  Convert : xbsIndex_r4/Convert  arith_type=Boolean  n_bits=16  bin_pt=14  quantization=Truncate  overflow=Wrap  latency=0  dbl_ovrd=off  pipeline=off  block_type=convert
      settings: block_version=10.1.3
  Convert1 : xbsIndex_r4/Convert  arith_type=Boolean  n_bits=16  bin_pt=14  quantization=Truncate  overflow=Wrap  latency=0  dbl_ovrd=off  pipeline=off  block_type=convert
      settings: block_version=10.1.3
  Register2 : xbsIndex_r4/Register  init=0  rst=on  en=on  dbl_ovrd=off  block_type=register
      settings: xl_area=[1 1 0 0 0 0 0]  block_version=VER_STRING_GOES_HERE
  Q : Outport  OutDataType=sfix(16)  OutScaling=2^0
  wires:
  Convert1/1 -> Register2/3
  Convert/1 -> Register2/2
  S/1 -> Convert1/1
  Register2/1 -> Q/1
  R/1 -> Convert/1
  Constant1/1 -> Register2/1

== system ofdm_txrx_supermimo/OFDM Rx MIMO/Equalizer & Packetizer/Packet_Constructor/Data Buffer/AutoResponse Processing/AutoResponse I/O/S-R Latch2 ==
  S : Inport  OutDataType=sfix(16)  OutScaling=2^0
  R : Inport  Port=2  OutDataType=sfix(16)  OutScaling=2^0
  Constant1 : xbsIndex_r4/Constant  arith_type=Boolean  const=1  n_bits=1  bin_pt=0  explicit_period=off  period=1  equ=P=C  opselect=C  inp2=PCIN>>17  opr=+  inp1=P  carry=CIN  dbl_ovrd=off  block_type=constant
      settings: block_version=VER_STRING_GOES_HERE
  Convert : xbsIndex_r4/Convert  arith_type=Boolean  n_bits=16  bin_pt=14  quantization=Truncate  overflow=Wrap  latency=0  dbl_ovrd=off  pipeline=off  block_type=convert
      settings: block_version=10.1.3
  Convert1 : xbsIndex_r4/Convert  arith_type=Boolean  n_bits=16  bin_pt=14  quantization=Truncate  overflow=Wrap  latency=0  dbl_ovrd=off  pipeline=off  block_type=convert
      settings: block_version=10.1.3
  Register2 : xbsIndex_r4/Register  init=0  rst=on  en=on  dbl_ovrd=off  block_type=register
      settings: xl_area=[1 1 0 0 0 0 0]  block_version=VER_STRING_GOES_HERE
  Q : Outport  OutDataType=sfix(16)  OutScaling=2^0
  wires:
  Constant1/1 -> Register2/1
  R/1 -> Convert/1
  Register2/1 -> Q/1
  S/1 -> Convert1/1
  Convert/1 -> Register2/2
  Convert1/1 -> Register2/3

== system ofdm_txrx_supermimo/OFDM Rx MIMO/Equalizer & Packetizer/Packet_Constructor/Data Buffer/AutoResponse Processing/AutoResponse I/O/S-R Latch3 ==
  S : Inport  OutDataType=sfix(16)  OutScaling=2^0
  R : Inport  Port=2  OutDataType=sfix(16)  OutScaling=2^0
  Constant1 : xbsIndex_r4/Constant  arith_type=Boolean  const=1  n_bits=1  bin_pt=0  explicit_period=off  period=1  equ=P=C  opselect=C  inp2=PCIN>>17  opr=+  inp1=P  carry=CIN  dbl_ovrd=off  block_type=constant
      settings: block_version=VER_STRING_GOES_HERE
  Convert : xbsIndex_r4/Convert  arith_type=Boolean  n_bits=16  bin_pt=14  quantization=Truncate  overflow=Wrap  latency=0  dbl_ovrd=off  pipeline=off  block_type=convert
      settings: block_version=10.1.3
  Convert1 : xbsIndex_r4/Convert  arith_type=Boolean  n_bits=16  bin_pt=14  quantization=Truncate  overflow=Wrap  latency=0  dbl_ovrd=off  pipeline=off  block_type=convert
      settings: block_version=10.1.3
  Register2 : xbsIndex_r4/Register  init=0  rst=on  en=on  dbl_ovrd=off  block_type=register
      settings: xl_area=[1 1 0 0 0 0 0]  block_version=VER_STRING_GOES_HERE
  Q : Outport  OutDataType=sfix(16)  OutScaling=2^0
  wires:
  Constant1/1 -> Register2/1
  R/1 -> Convert/1
  Register2/1 -> Q/1
  S/1 -> Convert1/1
  Convert/1 -> Register2/2
  Convert1/1 -> Register2/3

== system ofdm_txrx_supermimo/OFDM Rx MIMO/Equalizer & Packetizer/Packet_Constructor/Data Buffer/AutoResponse Processing/AutoResponse I/O/S-R Latch4 ==
  S : Inport  OutDataType=sfix(16)  OutScaling=2^0
  R : Inport  Port=2  OutDataType=sfix(16)  OutScaling=2^0
  Constant1 : xbsIndex_r4/Constant  arith_type=Boolean  const=1  n_bits=1  bin_pt=0  explicit_period=off  period=1  equ=P=C  opselect=C  inp2=PCIN>>17  opr=+  inp1=P  carry=CIN  dbl_ovrd=off  block_type=constant
      settings: block_version=VER_STRING_GOES_HERE
  Convert : xbsIndex_r4/Convert  arith_type=Boolean  n_bits=16  bin_pt=14  quantization=Truncate  overflow=Wrap  latency=0  dbl_ovrd=off  pipeline=off  block_type=convert
      settings: block_version=10.1.3
  Convert1 : xbsIndex_r4/Convert  arith_type=Boolean  n_bits=16  bin_pt=14  quantization=Truncate  overflow=Wrap  latency=0  dbl_ovrd=off  pipeline=off  block_type=convert
      settings: block_version=10.1.3
  Register2 : xbsIndex_r4/Register  init=0  rst=on  en=on  dbl_ovrd=off  block_type=register
      settings: xl_area=[1 1 0 0 0 0 0]  block_version=VER_STRING_GOES_HERE
  Q : Outport  OutDataType=sfix(16)  OutScaling=2^0
  wires:
  Convert1/1 -> Register2/3
  Convert/1 -> Register2/2
  S/1 -> Convert1/1
  Register2/1 -> Q/1
  R/1 -> Convert/1
  Constant1/1 -> Register2/1

== system ofdm_txrx_supermimo/OFDM Rx MIMO/Equalizer & Packetizer/Packet_Constructor/Data Buffer/AutoResponse Processing/AutoResponse I/O/Tx-Rx Generation ==
  note: RxEn can be asserted either by an automatic
      | Tx finishing, or by a register write. This allows
      | software to enabled Rx at boot, then hand over
      | control to the autoResponse subsystem.
  note: Give TxDone time to fall
      | before starting AutoTwoTx counter
  DoTx : Inport
  TxDone : Inport  Port=2
  Convert : xbsIndex_r4/Convert  arith_type=Boolean  n_bits=16  bin_pt=14  quantization=Truncate  overflow=Wrap  latency=0  dbl_ovrd=off  pipeline=off  block_type=convert
      settings: block_version=10.1.3
  Convert1 : xbsIndex_r4/Convert  arith_type=Boolean  n_bits=16  bin_pt=14  quantization=Truncate  overflow=Wrap  latency=0  dbl_ovrd=off  pipeline=off  block_type=convert
      settings: block_version=10.1.3
  Delay : Subsystem
  Delay2 : xbsIndex_r4/Delay  Orientation=up  en=off  latency=4  dbl_ovrd=off  reg_retiming=off  block_type=delay
      settings: infoedit=Hardware notes: A delay line is a chain, each link of which is an SRL16 followed by a flip-flop. If register retiming is enabled, the delay line is a chain of flip-flops.  xl_area=[1 1 0 0 0 0 0]  block_version=VER_STRING_GOES_HERE
  Ext_TxEn : Subsystem
  Logical1 : xbsIndex_r4/Logical  logical_function=OR  inputs=5  en=off  latency=0  precision=Full  arith_type=Unsigned  n_bits=16  bin_pt=0  align_bp=on  dbl_ovrd=off  block_type=logical
  Logical2 : xbsIndex_r4/Logical  logical_function=OR  inputs=2  en=off  latency=0  precision=Full  arith_type=Unsigned  n_bits=16  bin_pt=0  align_bp=on  dbl_ovrd=off  block_type=logical
  Logical3 : xbsIndex_r4/Logical  logical_function=AND  inputs=2  en=off  latency=0  precision=Full  arith_type=Unsigned  n_bits=16  bin_pt=0  align_bp=on  dbl_ovrd=off  block_type=logical
  Logical4 : xbsIndex_r4/Logical  logical_function=AND  inputs=2  en=off  latency=0  precision=Full  arith_type=Unsigned  n_bits=16  bin_pt=0  align_bp=on  dbl_ovrd=off  block_type=logical
  Logical5 : xbsIndex_r4/Logical  logical_function=OR  inputs=3  en=off  latency=0  precision=Full  arith_type=Unsigned  n_bits=16  bin_pt=0  align_bp=on  dbl_ovrd=off  block_type=logical
  Logical6 : xbsIndex_r4/Logical  logical_function=AND  inputs=2  en=off  latency=0  precision=Full  arith_type=Unsigned  n_bits=16  bin_pt=0  align_bp=on  dbl_ovrd=off  block_type=logical
  Logical7 : xbsIndex_r4/Logical  logical_function=OR  inputs=2  en=off  latency=0  precision=Full  arith_type=Unsigned  n_bits=16  bin_pt=0  align_bp=on  dbl_ovrd=off  block_type=logical
  Logical8 : xbsIndex_r4/Logical  Orientation=left  logical_function=OR  inputs=3  en=off  latency=0  precision=Full  arith_type=Unsigned  n_bits=16  bin_pt=0  align_bp=on  dbl_ovrd=off  block_type=logical
  S-R Latch1 : Subsystem
  S-R Latch2 : Subsystem
  S-R Latch3 : Subsystem
  S-R Latch4 : Subsystem
  S-R Latch5 : Subsystem
  Up Sample{,2..8,10} (9 alike) : xbsIndex_r4/Up Sample  sample_ratio=2  copy_samples=on  dbl_ovrd=off  block_type=usamp
      settings: infoedit="Up samples input data.  Inserted values  can be zeros or copies of the most recent input sample.<P><P>Hardware notes: No hardware is needed if inserted values are copies of the input sample; otherwise, a mux and single bit flip-flop are used."  block_version=10.1.3
  Up Sample1 : xbsIndex_r4/Up Sample  sample_ratio=4  copy_samples=on  dbl_ovrd=off  block_type=usamp
      settings: infoedit="Up samples input data.  Inserted values  can be zeros or copies of the most recent input sample.<P><P>Hardware notes: No hardware is needed if inserted values are copies of the input sample; otherwise, a mux and single bit flip-flop are used."  block_version=10.1.3
  posedge1 : Subsystem
  posedge2 : Subsystem
  ExtTxEn : Outport
  AutoTxEn : Outport  Port=2
  UserTxEn : Outport  Port=3
  RxEn : Outport  Port=4
  wires:
  [rx_global_reset] -> Up Sample2/1
  TxDone/1 -> Up Sample1/1
  S-R Latch2/1(Q) -> AutoTxEn/1
  S-R Latch1/1(Q) -> RxEn/1
  Logical5/1 -> S-R Latch2/2(R), S-R Latch3/2(R), S-R Latch5/2(R), Delay/3(Rst)
  [rx_global_reset] -> Up Sample5/1
  Logical1/1 -> S-R Latch1/2(R)
  [RxReg_radioRxEnable] -> Up Sample4/1
  Logical2/1 -> S-R Latch1/1(S)
  DoTx/1 -> Up Sample/1
  posedge1/1(Out) -> Logical4/2, S-R Latch3/1(S), Logical1/2
  S-R Latch3/1(Q) -> UserTxEn/1
  posedge2/1(Out) -> Delay/1(Start)
  [TxReset] -> Up Sample3/1
  [TxReset] -> Up Sample6/1
  Delay/1(TxEn) -> S-R Latch5/1(S), Logical1/1
  S-R Latch5/1(Q) -> ExtTxEn/1
  Up Sample/1 -> S-R Latch2/1(S), Logical1/3
  Up Sample2/1 -> Logical5/2, Logical8/3
  Up Sample3/1 -> Logical5/1, Logical8/2
  Up Sample4/1 -> Logical2/2
  Up Sample5/1 -> Logical1/4
  Up Sample6/1 -> Logical1/5
  Up Sample1/1 -> Logical2/1, Logical5/3, Logical6/2
  [regTx_AutoTwoTx_En] -> Convert1/1
  Convert1/1 -> Up Sample10/1
  Up Sample10/1 -> Logical4/1, Delay/2(En)
  [TxReg_TxStart] -> Up Sample7/1
  [TxReg_SoftTxStart_TxEn] -> Convert/1
  Logical3/1 -> posedge1/1(In)
  Convert/1 -> Up Sample8/1
  Up Sample7/1 -> Logical3/1
  Up Sample8/1 -> Logical3/2
  Logical4/1 -> S-R Latch4/1(S)
  S-R Latch4/1(Q) -> Logical6/1
  Logical7/1 -> posedge2/1(In)
  Ext_TxEn/1(TxEn) -> Logical7/1
  Logical6/1 -> Logical8/1, Delay2/1
  Logical8/1 -> S-R Latch4/2(R)
  Delay2/1 -> Logical7/2

== system ofdm_txrx_supermimo/OFDM Rx MIMO/Equalizer & Packetizer/Packet_Constructor/Data Buffer/AutoResponse Processing/AutoResponse I/O/negedge ==
  In : Inport  OutDataType=sfix(16)  OutScaling=2^0
  Delay : xbsIndex_r4/Delay  en=off  latency=1  dbl_ovrd=off  reg_retiming=off  block_type=delay
      settings: infoedit=Hardware notes: A delay line is a chain, each link of which is an SRL16 followed by a flip-flop. If register retiming is enabled, the delay line is a chain of flip-flops.  xl_area=[1 1 0 0 0 0 0]  block_version=8.2
  Inverter : xbsIndex_r4/Inverter  en=off  latency=0  dbl_ovrd=off  block_type=inv
      settings: xl_area=[0 0 0 0 0 0 0]  block_version=8.2
  Logical : xbsIndex_r4/Logical  logical_function=AND  inputs=2  en=off  latency=0  precision=Full  arith_type=Unsigned  n_bits=16  bin_pt=0  align_bp=on  dbl_ovrd=off  block_type=logical
      settings: xl_area=[1 0 0 1 0 0 0]  block_version=8.2
  Out : Outport  OutDataType=sfix(16)  OutScaling=2^0
  wires:
  Logical/1 -> Out/1
  In/1 -> Delay/1, Inverter/1
  Delay/1 -> Logical/2
  Inverter/1 -> Logical/1
